simulink model "CCMLoad"  (R2022b)
settings: Solver=FixedStepDiscrete  StartTime=0.0  StopTime=10.0  FixedStep=0.005  RelTol=1e-3
model workspace (MAT data in the model file):
  NvMBlockCarCfgBulkData : Simulink.Signal (value not decoded)
  NvMBlockCarCfgBulkDataInit : Simulink.Parameter (value not decoded)
symbols that are objects in the model workspace (value not decoded): NvMBlockCarCfgBulkDataInit
undefined symbols (not defined in the file or in its folder): DioChannelType, IcuChannelType, NvMBlockId, P_DioLevelType, P_Std_ReturnType, P_u16DutyCycle, PwmChannelType
(part 2 of 2)

== system CCMLoad/LoadDtcService/LoadDtcService_ReBlwMos_CtrFail_sys ==
  function : TriggerPort  TriggerType=function-call  IsSimulinkFunction=on  FunctionName=LoadDtcService_ReBlwMos_CtrFail  VariantControl=(inherit)
  Ground_1 : Ground
  Ground_2 : Ground
  PA_NvMBlockCarCfgBulkDataInit : Constant  Value=NvMBlockCarCfgBulkDataInit
  Terminator_1 : Terminator
  Retval : ArgOut  OutDataTypeStr=Enum: DTCState  ArgumentName=Retval
  ERR : ArgOut  Port=2  OutDataTypeStr=Std_ReturnType  ArgumentName=ERR
  wires:
  PA_NvMBlockCarCfgBulkDataInit/1 -> Terminator_1/1
  Ground_1/1 -> Retval/1
  Ground_2/1 -> ERR/1

== system CCMLoad/LoadDtcService/LoadDtcService_ReBlwPwm_CtrFail_sys ==
  function : TriggerPort  TriggerType=function-call  IsSimulinkFunction=on  FunctionName=LoadDtcService_ReBlwPwm_CtrFail  VariantControl=(inherit)
  Ground_1 : Ground
  Ground_2 : Ground
  PA_NvMBlockCarCfgBulkDataInit : Constant  Value=NvMBlockCarCfgBulkDataInit
  Terminator_1 : Terminator
  RetVal : ArgOut  OutDataTypeStr=Enum: DTCState  ArgumentName=RetVal
  ERR : ArgOut  Port=2  OutDataTypeStr=Std_ReturnType  ArgumentName=ERR
  wires:
  PA_NvMBlockCarCfgBulkDataInit/1 -> Terminator_1/1
  Ground_1/1 -> RetVal/1
  Ground_2/1 -> ERR/1

== system CCMLoad/LoadDtcService/LoadDtcService_ReTXV_OpenDtcStatus_sys ==
  function : TriggerPort  TriggerType=function-call  IsSimulinkFunction=on  FunctionName=LoadDtcService_ReTXV_OpenDtcStatus  VariantControl=(inherit)
  Ground_1 : Ground
  Ground_2 : Ground
  PA_NvMBlockCarCfgBulkDataInit : Constant  Value=NvMBlockCarCfgBulkDataInit
  Terminator_1 : Terminator
  Retval : ArgOut  OutDataTypeStr=Enum: DTCState  ArgumentName=Retval
  ERR : ArgOut  Port=2  OutDataTypeStr=Std_ReturnType  ArgumentName=ERR
  wires:
  PA_NvMBlockCarCfgBulkDataInit/1 -> Terminator_1/1
  Ground_1/1 -> Retval/1
  Ground_2/1 -> ERR/1

== system CCMLoad/LoadDtcService/LoadDtcService_ReTXV_ShortBatDtcStatus_sys ==
  function : TriggerPort  TriggerType=function-call  IsSimulinkFunction=on  FunctionName=LoadDtcService_ReTXV_ShortBatDtcStatus  VariantControl=(inherit)
  Ground_1 : Ground
  Ground_2 : Ground
  PA_NvMBlockCarCfgBulkDataInit : Constant  Value=NvMBlockCarCfgBulkDataInit
  Terminator_1 : Terminator
  Retval : ArgOut  OutDataTypeStr=Enum: DTCState  ArgumentName=Retval
  ERR : ArgOut  Port=2  OutDataTypeStr=Std_ReturnType  ArgumentName=ERR
  wires:
  PA_NvMBlockCarCfgBulkDataInit/1 -> Terminator_1/1
  Ground_1/1 -> Retval/1
  Ground_2/1 -> ERR/1

== system CCMLoad/LoadDtcService/LoadDtcService_VGAPowerFb_ShortBat_sys ==
  function : TriggerPort  TriggerType=function-call  IsSimulinkFunction=on  FunctionName=LoadDtcService_VGAPowerFb_ShortBat  VariantControl=(inherit)
  Ground_1 : Ground
  Ground_2 : Ground
  PA_NvMBlockCarCfgBulkDataInit : Constant  Value=NvMBlockCarCfgBulkDataInit
  Terminator_1 : Terminator
  RetVal : ArgOut  OutDataTypeStr=Enum: DTCState  ArgumentName=RetVal
  ERR : ArgOut  Port=2  OutDataTypeStr=Std_ReturnType  ArgumentName=ERR
  wires:
  PA_NvMBlockCarCfgBulkDataInit/1 -> Terminator_1/1
  Ground_1/1 -> RetVal/1
  Ground_2/1 -> ERR/1

== system CCMLoad/LoadDtcService/LoadDtcService_VGAPowerFb_ShortGnd_sys ==
  function : TriggerPort  TriggerType=function-call  IsSimulinkFunction=on  FunctionName=LoadDtcService_VGAPowerFb_ShortGnd  VariantControl=(inherit)
  Ground_1 : Ground
  Ground_2 : Ground
  PA_NvMBlockCarCfgBulkDataInit : Constant  Value=NvMBlockCarCfgBulkDataInit
  Terminator_1 : Terminator
  RetVal : ArgOut  OutDataTypeStr=Enum: DTCState  ArgumentName=RetVal
  ERR : ArgOut  Port=2  OutDataTypeStr=Std_ReturnType  ArgumentName=ERR
  wires:
  PA_NvMBlockCarCfgBulkDataInit/1 -> Terminator_1/1
  Ground_1/1 -> RetVal/1
  Ground_2/1 -> ERR/1

== system CCMLoad/LoadParaService/LoadParaService_FreezeIncarReqStatus_sys ==
  function : TriggerPort  TriggerType=function-call  IsSimulinkFunction=on  FunctionName=LoadParaService_FreezeIncarReqStatus  VariantControl=(inherit)
  Ground_1 : Ground
  PA_NvMBlockCarCfgBulkDataInit : Constant  Value=NvMBlockCarCfgBulkDataInit
  Terminator_1 : Terminator
  ERR : ArgOut  OutDataTypeStr=Std_ReturnType  ArgumentName=ERR
  wires:
  PA_NvMBlockCarCfgBulkDataInit/1 -> Terminator_1/1
  Ground_1/1 -> ERR/1

== system CCMLoad/LoadParaService/LoadParaService_FreezeReTXVReqStatus_sys ==
  function : TriggerPort  TriggerType=function-call  IsSimulinkFunction=on  FunctionName=LoadParaService_FreezeReTXVReqStatus  VariantControl=(inherit)
  Ground_1 : Ground
  PA_NvMBlockCarCfgBulkDataInit : Constant  Value=NvMBlockCarCfgBulkDataInit
  Terminator_1 : Terminator
  ERR : ArgOut  OutDataTypeStr=Std_ReturnType  ArgumentName=ERR
  wires:
  PA_NvMBlockCarCfgBulkDataInit/1 -> Terminator_1/1
  Ground_1/1 -> ERR/1

== system CCMLoad/LoadParaService/LoadParaService_FreezeVlclPwmPercent_sys ==
  function : TriggerPort  TriggerType=function-call  IsSimulinkFunction=on  FunctionName=LoadParaService_FreezeVlclPwmPercent  VariantControl=(inherit)
  Ground_1 : Ground
  PA_NvMBlockCarCfgBulkDataInit : Constant  Value=NvMBlockCarCfgBulkDataInit
  Terminator_1 : Terminator
  ERR : ArgOut  OutDataTypeStr=Std_ReturnType  ArgumentName=ERR
  wires:
  PA_NvMBlockCarCfgBulkDataInit/1 -> Terminator_1/1
  Ground_1/1 -> ERR/1

== system CCMLoad/LoadParaService/LoadParaService_FreezeVlclRearPwmPercent_sys ==
  function : TriggerPort  TriggerType=function-call  IsSimulinkFunction=on  FunctionName=LoadParaService_FreezeVlclRearPwmPercent  VariantControl=(inherit)
  Ground_1 : Ground
  PA_NvMBlockCarCfgBulkDataInit : Constant  Value=NvMBlockCarCfgBulkDataInit
  Terminator_1 : Terminator
  ERR : ArgOut  OutDataTypeStr=Std_ReturnType  ArgumentName=ERR
  wires:
  PA_NvMBlockCarCfgBulkDataInit/1 -> Terminator_1/1
  Ground_1/1 -> ERR/1

== system CCMLoad/LoadParaService/LoadParaService_IncarReqRetCtrltoECU_sys ==
  function : TriggerPort  TriggerType=function-call  IsSimulinkFunction=on  FunctionName=LoadParaService_IncarReqRetCtrltoECU  VariantControl=(inherit)
  Ground_1 : Ground
  PA_NvMBlockCarCfgBulkDataInit : Constant  Value=NvMBlockCarCfgBulkDataInit
  Terminator_1 : Terminator
  ERR : ArgOut  OutDataTypeStr=Std_ReturnType  ArgumentName=ERR
  wires:
  PA_NvMBlockCarCfgBulkDataInit/1 -> Terminator_1/1
  Ground_1/1 -> ERR/1

== system CCMLoad/LoadParaService/LoadParaService_IncarReqShortTermAdj_sys ==
  function : TriggerPort  TriggerType=function-call  IsSimulinkFunction=on  FunctionName=LoadParaService_IncarReqShortTermAdj  VariantControl=(inherit)
  Ground_1 : Ground
  PA_NvMBlockCarCfgBulkDataInit : Constant  Value=NvMBlockCarCfgBulkDataInit
  Terminator_1 : Terminator
  Terminator_2 : Terminator
  Status : ArgIn  OutDataTypeStr=UInt8  ArgumentName=Status
  ERR : ArgOut  OutDataTypeStr=Std_ReturnType  ArgumentName=ERR
  wires:
  PA_NvMBlockCarCfgBulkDataInit/1 -> Terminator_1/1
  Status/1 -> Terminator_2/1
  Ground_1/1 -> ERR/1

== system CCMLoad/LoadParaService/LoadParaService_ReTXVReqRetCtrltoECU_sys ==
  function : TriggerPort  TriggerType=function-call  IsSimulinkFunction=on  FunctionName=LoadParaService_ReTXVReqRetCtrltoECU  VariantControl=(inherit)
  Ground_1 : Ground
  PA_NvMBlockCarCfgBulkDataInit : Constant  Value=NvMBlockCarCfgBulkDataInit
  Terminator_1 : Terminator
  ERR : ArgOut  OutDataTypeStr=Std_ReturnType  ArgumentName=ERR
  wires:
  PA_NvMBlockCarCfgBulkDataInit/1 -> Terminator_1/1
  Ground_1/1 -> ERR/1

== system CCMLoad/LoadParaService/LoadParaService_ReTXVReqShortTermAdj_sys ==
  function : TriggerPort  TriggerType=function-call  IsSimulinkFunction=on  FunctionName=LoadParaService_ReTXVReqShortTermAdj  VariantControl=(inherit)
  Ground_1 : Ground
  PA_NvMBlockCarCfgBulkDataInit : Constant  Value=NvMBlockCarCfgBulkDataInit
  Terminator_1 : Terminator
  Terminator_2 : Terminator
  Status : ArgIn  OutDataTypeStr=UInt8  ArgumentName=Status
  ERR : ArgOut  OutDataTypeStr=Std_ReturnType  ArgumentName=ERR
  wires:
  PA_NvMBlockCarCfgBulkDataInit/1 -> Terminator_1/1
  Status/1 -> Terminator_2/1
  Ground_1/1 -> ERR/1

== system CCMLoad/LoadParaService/LoadParaService_ReadIncarMotorStatus_sys ==
  function : TriggerPort  TriggerType=function-call  IsSimulinkFunction=on  FunctionName=LoadParaService_ReadIncarMotorStatus  VariantControl=(inherit)
  Ground_1 : Ground
  Ground_2 : Ground
  PA_NvMBlockCarCfgBulkDataInit : Constant  Value=NvMBlockCarCfgBulkDataInit
  Terminator_1 : Terminator
  Status : ArgOut  OutDataTypeStr=UInt8  ArgumentName=Status
  ERR : ArgOut  Port=2  OutDataTypeStr=Std_ReturnType  ArgumentName=ERR
  wires:
  PA_NvMBlockCarCfgBulkDataInit/1 -> Terminator_1/1
  Ground_1/1 -> Status/1
  Ground_2/1 -> ERR/1

== system CCMLoad/LoadParaService/LoadParaService_ReadReTXVStatus_sys ==
  function : TriggerPort  TriggerType=function-call  IsSimulinkFunction=on  FunctionName=LoadParaService_ReadReTXVStatus  VariantControl=(inherit)
  Ground_1 : Ground
  Ground_2 : Ground
  PA_NvMBlockCarCfgBulkDataInit : Constant  Value=NvMBlockCarCfgBulkDataInit
  Terminator_1 : Terminator
  Status : ArgOut  OutDataTypeStr=UInt8  ArgumentName=Status
  ERR : ArgOut  Port=2  OutDataTypeStr=Std_ReturnType  ArgumentName=ERR
  wires:
  PA_NvMBlockCarCfgBulkDataInit/1 -> Terminator_1/1
  Ground_1/1 -> Status/1
  Ground_2/1 -> ERR/1

== system CCMLoad/LoadParaService/LoadParaService_SetBlwFbTriggerFlg_sys ==
  function : TriggerPort  TriggerType=function-call  IsSimulinkFunction=on  FunctionName=LoadParaService_SetBlwFbTriggerFlg  VariantControl=(inherit)
  Ground_1 : Ground
  PA_NvMBlockCarCfgBulkDataInit : Constant  Value=NvMBlockCarCfgBulkDataInit
  Terminator_1 : Terminator
  ERR : ArgOut  OutDataTypeStr=Std_ReturnType  ArgumentName=ERR
  wires:
  PA_NvMBlockCarCfgBulkDataInit/1 -> Terminator_1/1
  Ground_1/1 -> ERR/1

== system CCMLoad/LoadParaService/LoadParaService_VlclPwmRetCtrltoECU_sys ==
  function : TriggerPort  TriggerType=function-call  IsSimulinkFunction=on  FunctionName=LoadParaService_VlclPwmRetCtrltoECU  VariantControl=(inherit)
  Ground_1 : Ground
  PA_NvMBlockCarCfgBulkDataInit : Constant  Value=NvMBlockCarCfgBulkDataInit
  Terminator_1 : Terminator
  ERR : ArgOut  OutDataTypeStr=Std_ReturnType  ArgumentName=ERR
  wires:
  PA_NvMBlockCarCfgBulkDataInit/1 -> Terminator_1/1
  Ground_1/1 -> ERR/1

== system CCMLoad/LoadParaService/LoadParaService_VlclPwmShortTermAdj_sys ==
  function : TriggerPort  TriggerType=function-call  IsSimulinkFunction=on  FunctionName=LoadParaService_VlclPwmShortTermAdj  VariantControl=(inherit)
  Ground_1 : Ground
  PA_NvMBlockCarCfgBulkDataInit : Constant  Value=NvMBlockCarCfgBulkDataInit
  Terminator_1 : Terminator
  Terminator_2 : Terminator
  Duty : ArgIn  OutDataTypeStr=UInt8  ArgumentName=Duty
  ERR : ArgOut  OutDataTypeStr=Std_ReturnType  ArgumentName=ERR
  wires:
  PA_NvMBlockCarCfgBulkDataInit/1 -> Terminator_1/1
  Duty/1 -> Terminator_2/1
  Ground_1/1 -> ERR/1

== system CCMLoad/LoadParaService/LoadParaService_VlclReadBlwDuty_sys ==
  function : TriggerPort  TriggerType=function-call  IsSimulinkFunction=on  FunctionName=LoadParaService_VlclReadBlwDuty  VariantControl=(inherit)
  Ground_1 : Ground
  Ground_2 : Ground
  PA_NvMBlockCarCfgBulkDataInit : Constant  Value=NvMBlockCarCfgBulkDataInit
  Terminator_1 : Terminator
  Duty : ArgOut  OutDataTypeStr=UInt8  ArgumentName=Duty
  ERR : ArgOut  Port=2  OutDataTypeStr=Std_ReturnType  ArgumentName=ERR
  wires:
  PA_NvMBlockCarCfgBulkDataInit/1 -> Terminator_1/1
  Ground_1/1 -> Duty/1
  Ground_2/1 -> ERR/1

== system CCMLoad/LoadParaService/LoadParaService_VlclReadRearBlwDuty_sys ==
  function : TriggerPort  TriggerType=function-call  IsSimulinkFunction=on  FunctionName=LoadParaService_VlclReadRearBlwDuty  VariantControl=(inherit)
  Ground_1 : Ground
  Ground_2 : Ground
  PA_NvMBlockCarCfgBulkDataInit : Constant  Value=NvMBlockCarCfgBulkDataInit
  Terminator_1 : Terminator
  Duty : ArgOut  OutDataTypeStr=UInt8  ArgumentName=Duty
  ERR : ArgOut  Port=2  OutDataTypeStr=Std_ReturnType  ArgumentName=ERR
  wires:
  PA_NvMBlockCarCfgBulkDataInit/1 -> Terminator_1/1
  Ground_1/1 -> Duty/1
  Ground_2/1 -> ERR/1

== system CCMLoad/LoadParaService/LoadParaService_VlclRearPwmRetCtrltoECU_sys ==
  function : TriggerPort  TriggerType=function-call  IsSimulinkFunction=on  FunctionName=LoadParaService_VlclRearPwmRetCtrltoECU  VariantControl=(inherit)
  Ground_1 : Ground
  PA_NvMBlockCarCfgBulkDataInit : Constant  Value=NvMBlockCarCfgBulkDataInit
  Terminator_1 : Terminator
  ERR : ArgOut  OutDataTypeStr=Std_ReturnType  ArgumentName=ERR
  wires:
  PA_NvMBlockCarCfgBulkDataInit/1 -> Terminator_1/1
  Ground_1/1 -> ERR/1

== system CCMLoad/LoadParaService/LoadParaService_VlclRearPwmShortTermAdj_sys ==
  function : TriggerPort  TriggerType=function-call  IsSimulinkFunction=on  FunctionName=LoadParaService_VlclRearPwmShortTermAdj  VariantControl=(inherit)
  Ground_1 : Ground
  PA_NvMBlockCarCfgBulkDataInit : Constant  Value=NvMBlockCarCfgBulkDataInit
  Terminator_1 : Terminator
  Terminator_2 : Terminator
  Duty : ArgIn  OutDataTypeStr=UInt8  ArgumentName=Duty
  ERR : ArgOut  OutDataTypeStr=Std_ReturnType  ArgumentName=ERR
  wires:
  PA_NvMBlockCarCfgBulkDataInit/1 -> Terminator_1/1
  Duty/1 -> Terminator_2/1
  Ground_1/1 -> ERR/1
